simulink model "non_linear_MBS_final"  (R2021b)
settings: Solver=ode15s  StartTime=0  StopTime=30  FixedStep=.00001  RelTol=1e-3
values from neighbouring files (symbols this model uses):
  A_ratio = 1.41    [non_linear_param_MBS_PF_final.m]    (also in non_linear_param_final.m)
  Annulus_area = Bore_area-Rod_area    (= 2356.19449019)    [non_linear_param_MBS_PF_final.m]    (also in non_linear_param_final.m)
  beta_F = 12000e5    (= 1200000000)    [non_linear_param_MBS_PF_final.m]    (also in non_linear_param_final.m)
  Bore_area = pi/4*Bore_diameter^2    (= 3318.30724035)    [non_linear_param_MBS_PF_final.m]    (also in non_linear_param_final.m)
  cyl_c_end = 9e8    (= 900000000)    [non_linear_param_MBS_PF_final.m]    (also in non_linear_param_final.m)
  cyl_c_visc = 1500    [non_linear_param_MBS_PF_final.m]    (also in non_linear_param_final.m)
  cyl_CH_stat = 1/50    (= 0.02)    [non_linear_param_MBS_PF_final.m]    (also in non_linear_param_final.m)
  cyl_F_coul = 75    [non_linear_param_MBS_PF_final.m]    (also in non_linear_param_final.m)
  cyl_FH_stat = 500    [non_linear_param_MBS_PF_final.m]    (also in non_linear_param_final.m)
  cyl_gamma = 250    [non_linear_param_MBS_PF_final.m]    (also in non_linear_param_final.m)
  cyl_k_end = 6e9    (= 6000000000)    [non_linear_param_MBS_PF_final.m]    (also in non_linear_param_final.m)
  cyl_KLi = 0    [non_linear_param_MBS_PF_final.m]    (also in non_linear_param_final.m)
  d_eff_long = sqrt(2*J_sec_long*k_eff_long)    [non_linear_param_MBS_PF_final.m]    (also in non_linear_param_final.m)
  d_eff_short = sqrt(2*J_sec_short*k_eff_short)    [non_linear_param_MBS_PF_final.m]    (also in non_linear_param_final.m)
  DB = 0    [non_linear_param_MBS_PF_final.m]    (also in non_linear_param_final.m = -0.114)
  DR = 1    [non_linear_param_MBS_PF_final.m]    (also in non_linear_param_final.m)
  dr_dcv = 0.7    [non_linear_param_MBS_PF_final.m]    (also in non_linear_param_final.m)
  Eff_Bulk_On = 1    [non_linear_param_MBS_PF_final.m]    (also in non_linear_param_final.m)
  eta_air = 0.7e-2    (= 0.007)    [non_linear_param_MBS_PF_final.m]    (also in non_linear_param_final.m)
  k_cv = 15    [non_linear_param_MBS_PF_final.m]    (also in non_linear_param_final.m)
  k_DCV_PA = 7.85    [non_linear_param_MBS_PF_final.m]    (also in non_linear_param_final.m)
  k_DCV_PB = 7.55    [non_linear_param_MBS_PF_final.m]    (also in non_linear_param_final.m)
  k_DCV_T = 7.85    [non_linear_param_MBS_PF_final.m]    (also in non_linear_param_final.m)
  k_eff_long = E*I/I_sec_long    (= 3050381.94041)    [non_linear_param_MBS_PF_final.m]    (also in non_linear_param_final.m)
  k_eff_short = E*I/I_sec_short    (= 13451432.5427)    [non_linear_param_MBS_PF_final.m]    (also in non_linear_param_final.m)
  k_ocv = 15    [non_linear_param_MBS_PF_final.m]    (also in non_linear_param_final.m)
  K_oil = 1.5e4*0+12000    (= 12000)    [non_linear_param_MBS_PF_final.m]    (also in non_linear_param_final.m)
  k_tanh = 0.005    [non_linear_param_MBS_PF_final.m]    (also in non_linear_param_final.m)
  kappa_air = 1.4    [non_linear_param_MBS_PF_final.m]    (also in non_linear_param_final.m)
  KR = 1    [non_linear_param_MBS_PF_final.m]    (also in non_linear_param_final.m)
  mu_ocv = 10    [non_linear_param_MBS_PF_final.m]    (also in non_linear_param_final.m = 3)
  OL_pa = -0.114    [non_linear_param_MBS_PF_final.m]    (also in non_linear_param_final.m)
  OL_pb = 0.114    [non_linear_param_MBS_PF_final.m]    (also in non_linear_param_final.m)
  p0_A = 62.79    [non_linear_param_MBS_PF_final.m]    (also in non_linear_param_final.m)
  p0_A1 = 1.08    [non_linear_param_MBS_PF_final.m]    (also in non_linear_param_final.m)
  p0_B = 1.213    [non_linear_param_MBS_PF_final.m]    (also in non_linear_param_final.m)
  p_cv_fo = 1    [non_linear_param_MBS_PF_final.m]    (also in non_linear_param_final.m)
  p_cv_set = 2.8    [non_linear_param_MBS_PF_final.m]    (also in non_linear_param_final.m)
  p_ocv_fo = 350    [non_linear_param_MBS_PF_final.m]    (also in non_linear_param_final.m)
  p_ocv_set = 196    [non_linear_param_MBS_PF_final.m]    (also in non_linear_param_final.m = 150)
  p_pc_set = 7    [non_linear_param_MBS_PF_final.m]    (also in non_linear_param_final.m)
  p_R = 1.1    [non_linear_param_MBS_PF_final.m]    (also in non_linear_param_final.m)
  p_S = 180    [non_linear_param_MBS_PF_final.m]    (also in non_linear_param_final.m)
  patm = 1.013e5    (= 101300)    [non_linear_param_MBS_PF_final.m]    (also in non_linear_param_final.m)
  Stroke = 500    [non_linear_param_MBS_PF_final.m]    (also in non_linear_param_final.m)
  t0 = 3    [non_linear_param_MBS_PF_final.m]    (also in non_linear_param_final.m = 1)
  tau_ocv = 0.0889*0+0.001    (= 0.001)    [non_linear_param_MBS_PF_final.m]    (also in non_linear_param_final.m)
  tau_pc = tau_ocv    (= 0.001)    [non_linear_param_MBS_PF_final.m]    (also in non_linear_param_final.m)
  tStepDown = 4.7*0+7.5    (= 7.5)    [non_linear_param_MBS_PF_final.m]    (also in non_linear_param_final.m = 4.6)
  tStepUp = 10.0    (= 10)    [non_linear_param_MBS_PF_final.m]    (also in non_linear_param_final.m = 6.2)
  tWait = 5.0    (= 5)    [non_linear_param_MBS_PF_final.m]    (also in non_linear_param_final.m = 8)
  UL_at = -0.05    [non_linear_param_MBS_PF_final.m]    (also in non_linear_param_final.m)
  UL_bt = 0.05    [non_linear_param_MBS_PF_final.m]    (also in non_linear_param_final.m)
  Up = 1    [non_linear_param_MBS_PF_final.m]    (also in non_linear_param_final.m)
  uS_sp = -0.4    [non_linear_param_MBS_PF_final.m]    (also in non_linear_param_final.m = -0.55)
  V_CH_A = 1.0e-03    (= 0.001)    [non_linear_param_MBS_PF_final.m]    (also in non_linear_param_final.m)
  V_CH_A1 = 0.1e-03    (= 0.0001)    [non_linear_param_MBS_PF_final.m]    (also in non_linear_param_final.m)
  V_CH_B = 1.25e-03    (= 0.00125)    [non_linear_param_MBS_PF_final.m]    (also in non_linear_param_final.m)
  wn_dcv = 30    [non_linear_param_MBS_PF_final.m]    (also in non_linear_param_final.m)
undefined symbols (not defined in the file or in its folder): sigbuilder_block, smiData, uS0, X, Y, Z
libraries: sm_lib x189, nesl_utility x4, eeGeneralControl x2
(part 4 of 6)

== system non_linear_MBS_final/Test/CB_1 ==
  CB_850_1_RIGID : Subsystem
  CB_850_2_RIGID : Subsystem
  CB_plate_1_RIGID : Subsystem
  ReferenceFrame : sm_lib/Frames and Transforms/Reference Frame
  Transform : sm_lib/Frames and Transforms/Rigid Transform  TranslationMethod=Cartesian  TranslationStandardOffset=0  TranslationLengthUnit=mm  TranslationStandardAxis=+Z  TranslationCartesianOffset=smiData.RigidTransform(13).translation  TranslationROffset=0  TranslationROffsetUnits=m  TranslationZOffset=0  TranslationZOffsetUnits=m  TranslationThetaOffset=0  TranslationThetaOffsetUnits=deg  RotationMethod=ArbitraryAxis  RotationAngleUnits=rad  RotationStandardAxis=+Z  RotationAngle=smiData.RigidTransform(13).angle  RotationArbitraryAxis=smiData.RigidTransform(13).axis  FollAlignAxisA=+X  BaseAlignAxisA=+Y  FollAlignAxisB=+Y  BaseAlignAxisB=+Z  RotationSequenceAxes=FollowerAxes  RotationSequence=XYX  RotationSequenceAngles=[0 0 0]  RotationSequenceAnglesUnits=deg  RotationMatrix=[1 0 0; 0 1 0; 0 0 1]
  Transform1 : sm_lib/Frames and Transforms/Rigid Transform  TranslationMethod=Cartesian  TranslationStandardOffset=0  TranslationLengthUnit=mm  TranslationStandardAxis=+Z  TranslationCartesianOffset=smiData.RigidTransform(16).translation  TranslationROffset=0  TranslationROffsetUnits=m  TranslationZOffset=0  TranslationZOffsetUnits=m  TranslationThetaOffset=0  TranslationThetaOffsetUnits=deg  RotationMethod=ArbitraryAxis  RotationAngleUnits=rad  RotationStandardAxis=+Z  RotationAngle=smiData.RigidTransform(16).angle  RotationArbitraryAxis=smiData.RigidTransform(16).axis  FollAlignAxisA=+X  BaseAlignAxisA=+Y  FollAlignAxisB=+Y  BaseAlignAxisB=+Z  RotationSequenceAxes=FollowerAxes  RotationSequence=XYX  RotationSequenceAngles=[0 0 0]  RotationSequenceAnglesUnits=deg  RotationMatrix=[1 0 0; 0 1 0; 0 0 1]
  Transform3 : sm_lib/Frames and Transforms/Rigid Transform  TranslationMethod=Cartesian  TranslationStandardOffset=0  TranslationLengthUnit=mm  TranslationStandardAxis=+Z  TranslationCartesianOffset=smiData.RigidTransform(58).translation  TranslationROffset=0  TranslationROffsetUnits=m  TranslationZOffset=0  TranslationZOffsetUnits=m  TranslationThetaOffset=0  TranslationThetaOffsetUnits=deg  RotationMethod=ArbitraryAxis  RotationAngleUnits=rad  RotationStandardAxis=+Z  RotationAngle=smiData.RigidTransform(58).angle  RotationArbitraryAxis=smiData.RigidTransform(58).axis  FollAlignAxisA=+X  BaseAlignAxisA=+Y  FollAlignAxisB=+Y  BaseAlignAxisB=+Z  RotationSequenceAxes=FollowerAxes  RotationSequence=XYX  RotationSequenceAngles=[0 0 0]  RotationSequenceAnglesUnits=deg  RotationMatrix=[1 0 0; 0 1 0; 0 0 1]
  Transform4 : sm_lib/Frames and Transforms/Rigid Transform  TranslationMethod=Cartesian  TranslationStandardOffset=0  TranslationLengthUnit=mm  TranslationStandardAxis=+Z  TranslationCartesianOffset=smiData.RigidTransform(59).translation  TranslationROffset=0  TranslationROffsetUnits=m  TranslationZOffset=0  TranslationZOffsetUnits=m  TranslationThetaOffset=0  TranslationThetaOffsetUnits=deg  RotationMethod=ArbitraryAxis  RotationAngleUnits=rad  RotationStandardAxis=+Z  RotationAngle=smiData.RigidTransform(59).angle  RotationArbitraryAxis=smiData.RigidTransform(59).axis  FollAlignAxisA=+X  BaseAlignAxisA=+Y  FollAlignAxisB=+Y  BaseAlignAxisB=+Z  RotationSequenceAxes=FollowerAxes  RotationSequence=XYX  RotationSequenceAngles=[0 0 0]  RotationSequenceAnglesUnits=deg  RotationMatrix=[1 0 0; 0 1 0; 0 0 1]
  Transform5 : sm_lib/Frames and Transforms/Rigid Transform  TranslationMethod=Cartesian  TranslationStandardOffset=0  TranslationLengthUnit=mm  TranslationStandardAxis=+Z  TranslationCartesianOffset=smiData.RigidTransform(60).translation  TranslationROffset=0  TranslationROffsetUnits=m  TranslationZOffset=0  TranslationZOffsetUnits=m  TranslationThetaOffset=0  TranslationThetaOffsetUnits=deg  RotationMethod=ArbitraryAxis  RotationAngleUnits=rad  RotationStandardAxis=+Z  RotationAngle=smiData.RigidTransform(60).angle  RotationArbitraryAxis=smiData.RigidTransform(60).axis  FollAlignAxisA=+X  BaseAlignAxisA=+Y  FollAlignAxisB=+Y  BaseAlignAxisB=+Z  RotationSequenceAxes=FollowerAxes  RotationSequence=XYX  RotationSequenceAngles=[0 0 0]  RotationSequenceAnglesUnits=deg  RotationMatrix=[1 0 0; 0 1 0; 0 0 1]
  F1 : PMIOPort  Side=Left
  F : PMIOPort  Port=2  Side=Right
  connections:
  Transform/LConn1 -- ReferenceFrame/RConn1 -- Transform4/LConn1 -- Transform5/LConn1 -- Transform3/LConn1 -- Transform1/LConn1
  Transform/RConn1 -- F/RConn1
  Transform1/RConn1 -- F1/RConn1
  CB_850_1_RIGID/LConn1 -- Transform3/RConn1
  CB_850_2_RIGID/LConn1 -- Transform4/RConn1
  CB_plate_1_RIGID/LConn1 -- Transform5/RConn1

== system non_linear_MBS_final/Test/CB_2 ==
  CB_850_1_RIGID : Subsystem
  CB_850_2_RIGID : Subsystem
  CB_plate_1_RIGID : Subsystem
  ReferenceFrame : sm_lib/Frames and Transforms/Reference Frame
  Rigid Transform4 : sm_lib/Frames and Transforms/Rigid Transform  TranslationMethod=Cartesian  TranslationStandardOffset=0  TranslationLengthUnit=mm  TranslationStandardAxis=+Z  TranslationCartesianOffset=[0 0 -10]  TranslationROffset=0  TranslationROffsetUnits=m  TranslationZOffset=0  TranslationZOffsetUnits=m  TranslationThetaOffset=0  TranslationThetaOffsetUnits=deg  RotationMethod=ArbitraryAxis  RotationAngleUnits=deg  RotationStandardAxis=+Z  RotationAngle=90  RotationArbitraryAxis=[0 0 1]  FollAlignAxisA=+X  BaseAlignAxisA=+Y  FollAlignAxisB=+Y  BaseAlignAxisB=+Z  RotationSequenceAxes=FollowerAxes  RotationSequence=XYX  RotationSequenceAngles=[0 0 0]  RotationSequenceAnglesUnits=deg  RotationMatrix=[1 0 0; 0 1 0; 0 0 1]
  Transform : sm_lib/Frames and Transforms/Rigid Transform  TranslationMethod=Cartesian  TranslationStandardOffset=0  TranslationLengthUnit=mm  TranslationStandardAxis=+Z  TranslationCartesianOffset=smiData.RigidTransform(20).translation  TranslationROffset=0  TranslationROffsetUnits=m  TranslationZOffset=0  TranslationZOffsetUnits=m  TranslationThetaOffset=0  TranslationThetaOffsetUnits=deg  RotationMethod=ArbitraryAxis  RotationAngleUnits=rad  RotationStandardAxis=+Z  RotationAngle=smiData.RigidTransform(20).angle  RotationArbitraryAxis=smiData.RigidTransform(20).axis  FollAlignAxisA=+X  BaseAlignAxisA=+Y  FollAlignAxisB=+Y  BaseAlignAxisB=+Z  RotationSequenceAxes=FollowerAxes  RotationSequence=XYX  RotationSequenceAngles=[0 0 0]  RotationSequenceAnglesUnits=deg  RotationMatrix=[1 0 0; 0 1 0; 0 0 1]
  Transform3 : sm_lib/Frames and Transforms/Rigid Transform  TranslationMethod=Cartesian  TranslationStandardOffset=0  TranslationLengthUnit=mm  TranslationStandardAxis=+Z  TranslationCartesianOffset=smiData.RigidTransform(61).translation  TranslationROffset=0  TranslationROffsetUnits=m  TranslationZOffset=0  TranslationZOffsetUnits=m  TranslationThetaOffset=0  TranslationThetaOffsetUnits=deg  RotationMethod=ArbitraryAxis  RotationAngleUnits=rad  RotationStandardAxis=+Z  RotationAngle=smiData.RigidTransform(61).angle  RotationArbitraryAxis=smiData.RigidTransform(61).axis  FollAlignAxisA=+X  BaseAlignAxisA=+Y  FollAlignAxisB=+Y  BaseAlignAxisB=+Z  RotationSequenceAxes=FollowerAxes  RotationSequence=XYX  RotationSequenceAngles=[0 0 0]  RotationSequenceAnglesUnits=deg  RotationMatrix=[1 0 0; 0 1 0; 0 0 1]
  Transform4 : sm_lib/Frames and Transforms/Rigid Transform  TranslationMethod=Cartesian  TranslationStandardOffset=0  TranslationLengthUnit=mm  TranslationStandardAxis=+Z  TranslationCartesianOffset=smiData.RigidTransform(62).translation  TranslationROffset=0  TranslationROffsetUnits=m  TranslationZOffset=0  TranslationZOffsetUnits=m  TranslationThetaOffset=0  TranslationThetaOffsetUnits=deg  RotationMethod=ArbitraryAxis  RotationAngleUnits=rad  RotationStandardAxis=+Z  RotationAngle=smiData.RigidTransform(62).angle  RotationArbitraryAxis=smiData.RigidTransform(62).axis  FollAlignAxisA=+X  BaseAlignAxisA=+Y  FollAlignAxisB=+Y  BaseAlignAxisB=+Z  RotationSequenceAxes=FollowerAxes  RotationSequence=XYX  RotationSequenceAngles=[0 0 0]  RotationSequenceAnglesUnits=deg  RotationMatrix=[1 0 0; 0 1 0; 0 0 1]
  Transform5 : sm_lib/Frames and Transforms/Rigid Transform  TranslationMethod=Cartesian  TranslationStandardOffset=0  TranslationLengthUnit=mm  TranslationStandardAxis=+Z  TranslationCartesianOffset=smiData.RigidTransform(63).translation  TranslationROffset=0  TranslationROffsetUnits=m  TranslationZOffset=0  TranslationZOffsetUnits=m  TranslationThetaOffset=0  TranslationThetaOffsetUnits=deg  RotationMethod=ArbitraryAxis  RotationAngleUnits=rad  RotationStandardAxis=+Z  RotationAngle=smiData.RigidTransform(63).angle  RotationArbitraryAxis=smiData.RigidTransform(63).axis  FollAlignAxisA=+X  BaseAlignAxisA=+Y  FollAlignAxisB=+Y  BaseAlignAxisB=+Z  RotationSequenceAxes=FollowerAxes  RotationSequence=XYX  RotationSequenceAngles=[0 0 0]  RotationSequenceAnglesUnits=deg  RotationMatrix=[1 0 0; 0 1 0; 0 0 1]
  F1 : PMIOPort  Side=Left
  F : PMIOPort  Port=2  Side=Left
  connections:
  Transform/LConn1 -- Rigid Transform4/LConn1 -- ReferenceFrame/RConn1 -- Transform4/LConn1 -- Transform5/LConn1 -- Transform3/LConn1
  Transform/RConn1 -- F/RConn1
  Rigid Transform4/RConn1 -- F1/RConn1
  CB_850_1_RIGID/LConn1 -- Transform3/RConn1
  CB_plate_1_RIGID/LConn1 -- Transform4/RConn1
  CB_850_2_RIGID/LConn1 -- Transform5/RConn1

== system non_linear_MBS_final/Test/Cylinder Barrel ==
  ReferenceFrame : sm_lib/Frames and Transforms/Reference Frame
  Solid : sm_lib/Body Elements/File Solid  ExtGeomFileName=Cyl_hus_part_Default_sldprt.STEP  UnitType=FromFile  ExtGeomFileUnits=m  StepReaderType=OCC  ExportConvexHullGeometry=off  InertiaType=CalculateFromGeometry  Mass=smiData.Solid(20).mass  MassUnits=kg  CenterOfMass=smiData.Solid(20).CoM  CenterOfMassUnits=mm  MomentsOfInertia=smiData.Solid(20).MoI  MomentsOfInertiaUnits=kg*mm^2  ProductsOfInertia=smiData.Solid(20).PoI  ProductsOfInertiaUnits=kg*mm^2  BasedOnType=Density  Density=8702  DensityUnits=kg/m^3  DoExposeReferenceFrame=on  SerializedFrames=<Frames/>  GraphicType=FromGeometry  MarkerShape=Sphere  MarkerSize=10  GraphicVisPropType=SimpleVisualProperties  GraphicDiffuseColor=[0.0 0.6 1.0]  GraphicSpecularColor=[0.5 0.5 0.5 1.0]  GraphicAmbientColor=[0.15 0.15 0.15 1.0]  GraphicEmissiveColor=[0.0 0.0 0.0 1.0]  GraphicShininess=75  GraphicOpacity=smiData.Solid(20).opacity
  Transform : sm_lib/Frames and Transforms/Rigid Transform  TranslationMethod=Cartesian  TranslationStandardOffset=0  TranslationLengthUnit=mm  TranslationStandardAxis=+Z  TranslationCartesianOffset=smiData.RigidTransform(1).translation  TranslationROffset=0  TranslationROffsetUnits=m  TranslationZOffset=0  TranslationZOffsetUnits=m  TranslationThetaOffset=0  TranslationThetaOffsetUnits=deg  RotationMethod=ArbitraryAxis  RotationAngleUnits=rad  RotationStandardAxis=+Z  RotationAngle=smiData.RigidTransform(1).angle  RotationArbitraryAxis=smiData.RigidTransform(1).axis  FollAlignAxisA=+X  BaseAlignAxisA=+Y  FollAlignAxisB=+Y  BaseAlignAxisB=+Z  RotationSequenceAxes=FollowerAxes  RotationSequence=XYX  RotationSequenceAngles=[0 0 0]  RotationSequenceAnglesUnits=deg  RotationMatrix=[1 0 0; 0 1 0; 0 0 1]
  Transform1 : sm_lib/Frames and Transforms/Rigid Transform  TranslationMethod=Cartesian  TranslationStandardOffset=0  TranslationLengthUnit=mm  TranslationStandardAxis=+Z  TranslationCartesianOffset=smiData.RigidTransform(14).translation  TranslationROffset=0  TranslationROffsetUnits=m  TranslationZOffset=0  TranslationZOffsetUnits=m  TranslationThetaOffset=0  TranslationThetaOffsetUnits=deg  RotationMethod=ArbitraryAxis  RotationAngleUnits=rad  RotationStandardAxis=+Z  RotationAngle=smiData.RigidTransform(14).angle  RotationArbitraryAxis=smiData.RigidTransform(14).axis  FollAlignAxisA=+X  BaseAlignAxisA=+Y  FollAlignAxisB=+Y  BaseAlignAxisB=+Z  RotationSequenceAxes=FollowerAxes  RotationSequence=XYX  RotationSequenceAngles=[0 0 0]  RotationSequenceAnglesUnits=deg  RotationMatrix=[1 0 0; 0 1 0; 0 0 1]
  F1 : PMIOPort  Side=Left
  F : PMIOPort  Port=2  Side=Right
  connections:
  Solid/RConn1 -- ReferenceFrame/RConn1 -- Transform/LConn1 -- Transform1/LConn1
  Transform/RConn1 -- F/RConn1
  Transform1/RConn1 -- F1/RConn1

== system non_linear_MBS_final/Test/Cylinder Rod ==
  ReferenceFrame : sm_lib/Frames and Transforms/Reference Frame
  Solid : sm_lib/Body Elements/File Solid  ExtGeomFileName=Cyl_rod_part_Default_sldprt.STEP  UnitType=FromFile  ExtGeomFileUnits=m  StepReaderType=OCC  ExportConvexHullGeometry=off  InertiaType=CalculateFromGeometry  Mass=5  MassUnits=kg  CenterOfMass=smiData.Solid(1).CoM  CenterOfMassUnits=mm  MomentsOfInertia=smiData.Solid(1).MoI  MomentsOfInertiaUnits=kg*mm^2  ProductsOfInertia=smiData.Solid(1).PoI  ProductsOfInertiaUnits=kg*mm^2  BasedOnType=Mass  Density=1000  DensityUnits=kg/m^3  DoExposeReferenceFrame=on  SerializedFrames=<Frames/>  GraphicType=FromGeometry  MarkerShape=Sphere  MarkerSize=10  GraphicVisPropType=SimpleVisualProperties  GraphicDiffuseColor=smiData.Solid(1).color  GraphicSpecularColor=[0.5 0.5 0.5 1.0]  GraphicAmbientColor=[0.15 0.15 0.15 1.0]  GraphicEmissiveColor=[0.0 0.0 0.0 1.0]  GraphicShininess=75  GraphicOpacity=smiData.Solid(1).opacity
  Transform : sm_lib/Frames and Transforms/Rigid Transform  TranslationMethod=Cartesian  TranslationStandardOffset=0  TranslationLengthUnit=mm  TranslationStandardAxis=+Z  TranslationCartesianOffset=[2.4829027722717001e-12 (-50.259544455820055) 5.8920380567815703e-15]  TranslationROffset=0  TranslationROffsetUnits=m  TranslationZOffset=0  TranslationZOffsetUnits=m  TranslationThetaOffset=0  TranslationThetaOffsetUnits=deg  RotationMethod=ArbitraryAxis  RotationAngleUnits=rad  RotationStandardAxis=+Z  RotationAngle=smiData.RigidTransform(2).angle  RotationArbitraryAxis=smiData.RigidTransform(2).axis  FollAlignAxisA=+X  BaseAlignAxisA=+Y  FollAlignAxisB=+Y  BaseAlignAxisB=+Z  RotationSequenceAxes=FollowerAxes  RotationSequence=XYX  RotationSequenceAngles=[0 0 0]  RotationSequenceAnglesUnits=deg  RotationMatrix=[1 0 0; 0 1 0; 0 0 1]
  Transform1 : sm_lib/Frames and Transforms/Rigid Transform  TranslationMethod=Cartesian  TranslationStandardOffset=0  TranslationLengthUnit=mm  TranslationStandardAxis=+Z  TranslationCartesianOffset=smiData.RigidTransform(19).translation  TranslationROffset=0  TranslationROffsetUnits=m  TranslationZOffset=0  TranslationZOffsetUnits=m  TranslationThetaOffset=0  TranslationThetaOffsetUnits=deg  RotationMethod=ArbitraryAxis  RotationAngleUnits=rad  RotationStandardAxis=+Z  RotationAngle=smiData.RigidTransform(19).angle  RotationArbitraryAxis=smiData.RigidTransform(19).axis  FollAlignAxisA=+X  BaseAlignAxisA=+Y  FollAlignAxisB=+Y  BaseAlignAxisB=+Z  RotationSequenceAxes=FollowerAxes  RotationSequence=XYX  RotationSequenceAngles=[0 0 0]  RotationSequenceAnglesUnits=deg  RotationMatrix=[1 0 0; 0 1 0; 0 0 1]
  F : PMIOPort  Side=Left
  F1 : PMIOPort  Port=2  Side=Right
  connections:
  Solid/RConn1 -- ReferenceFrame/RConn1 -- Transform/LConn1 -- Transform1/LConn1
  Transform1/RConn1 -- F1/RConn1
  Transform/RConn1 -- F/RConn1

== system non_linear_MBS_final/Directional Control Valve/Edge Flows/Flow Characteristics ==
  u_B-T [-] : Inport
  p_B - p_R [bar] : Inport  Port=2
  Abs : Abs
  Constant : Constant  Value=k_DCV_T
  Product6 : Product  Inputs=4  RndMeth=Zero
  Sign : Subsystem
  Sqrt7 : Sqrt  SaturateOnIntegerOverflow=on
  Q_B-T [L/min] : Outport  VectorParamsAs1DForOutWhenUnconnected=off
  wires:
  Sqrt7/1 -> Product6/3  "[bar]^0.5"
  Product6/1 -> Q_B-T [L/min]/1  "[L/min]"
  p_B - p_R [bar]/1 -> Sign/1(In), Abs/1  "[bar]"
  Abs/1 -> Sqrt7/1
  u_B-T [-]/1 -> Product6/2  "[-]"
  Constant/1 -> Product6/1  "[L/min*bar^0.5]"
  Sign/1(Out) -> Product6/4

== system non_linear_MBS_final/Directional Control Valve/Edge Flows/Flow Characteristics1 ==
  u_A-T [-] : Inport
  p_A1 - p_R [bar] : Inport  Port=2
  Abs : Abs
  Constant : Constant  Value=k_DCV_T
  Product6 : Product  Inputs=4  RndMeth=Zero
  Sign : Subsystem
  Sqrt7 : Sqrt  SaturateOnIntegerOverflow=on
  Q_A-T [L/min] : Outport  VectorParamsAs1DForOutWhenUnconnected=off
  wires:
  Sqrt7/1 -> Product6/3  "[bar]^0.5"
  Product6/1 -> Q_A-T [L/min]/1  "[L/min]"
  p_A1 - p_R [bar]/1 -> Sign/1(In), Abs/1  "[bar]"
  Abs/1 -> Sqrt7/1
  u_A-T [-]/1 -> Product6/2  "[-]"
  Sign/1(Out) -> Product6/4
  Constant/1 -> Product6/1  "[L/min*bar^0.5]"

== system non_linear_MBS_final/Directional Control Valve/Edge Flows/Flow Characteristics2 ==
  u_P-A [-] : Inport
  p_C - p_A1 [bar] : Inport  Port=2
  Abs : Abs
  Constant : Constant  Value=k_DCV_PA
  Product6 : Product  Inputs=4  RndMeth=Zero
  Sign : Subsystem
  Sqrt7 : Sqrt  SaturateOnIntegerOverflow=on
  Q_P-A [L/min] : Outport  VectorParamsAs1DForOutWhenUnconnected=off
  wires:
  Sqrt7/1 -> Product6/3  "[bar]^0.5"
  Product6/1 -> Q_P-A [L/min]/1  "[L/min]"
  p_C - p_A1 [bar]/1 -> Sign/1(In), Abs/1  "[bar]"
  Abs/1 -> Sqrt7/1
  u_P-A [-]/1 -> Product6/2  "[-]"
  Sign/1(Out) -> Product6/4
  Constant/1 -> Product6/1  "[L/min*bar^0.5]"

== system non_linear_MBS_final/Directional Control Valve/Edge Flows/Flow Characteristics3 ==
  u_P-B [-] : Inport
  p_C - p_B [bar] : Inport  Port=2
  Abs : Abs
  Constant : Constant  Value=k_DCV_PB
  Product6 : Product  Inputs=4  RndMeth=Zero
  Sign : Subsystem
  Sqrt7 : Sqrt  SaturateOnIntegerOverflow=on
  Q_P-B [L/min] : Outport  VectorParamsAs1DForOutWhenUnconnected=off
  wires:
  Sqrt7/1 -> Product6/3  "[bar]^0.5"
  Product6/1 -> Q_P-B [L/min]/1  "[L/min]"
  p_C - p_B [bar]/1 -> Sign/1(In), Abs/1  "[bar]"
  Abs/1 -> Sqrt7/1
  u_P-B [-]/1 -> Product6/2  "[-]"
  Sign/1(Out) -> Product6/4
  Constant/1 -> Product6/1  "[L/min*bar^0.5]"

== system non_linear_MBS_final/Directional Control Valve/Edge Flows/Q_2 selection ==
  Q_P-A [l/min] : Inport
  Q_P-B [l/min] : Inport  Port=2
  Sum : Sum
  Q_DCV_In [l/min] : Outport  VectorParamsAs1DForOutWhenUnconnected=off
  wires:
  Sum/1 -> Q_DCV_In [l/min]/1
  Q_P-B [l/min]/1 -> Sum/2
  Q_P-A [l/min]/1 -> Sum/1

== system non_linear_MBS_final/Directional Control Valve/Edge Openings/Edge A-T ==
  u_dcv [-] : Inport
  MATLAB Function1 : MATLAB Function  TreatAsAtomicUnit=on
  u_A-T [-] : Outport  VectorParamsAs1DForOutWhenUnconnected=off
  wires:
  MATLAB Function1/1(u_AT) -> u_A-T [-]/1
  u_dcv [-]/1 -> MATLAB Function1/1(u_dcv)

== system non_linear_MBS_final/Directional Control Valve/Edge Openings/Edge B-T ==
  u_dcv [-] : Inport
  MATLAB Function : MATLAB Function  TreatAsAtomicUnit=on
  u_B-T [-] : Outport  VectorParamsAs1DForOutWhenUnconnected=off
  wires:
  u_dcv [-]/1 -> MATLAB Function/1(u_dcv)
  MATLAB Function/1(u_BT) -> u_B-T [-]/1

== system non_linear_MBS_final/Directional Control Valve/Edge Openings/Edge P-A ==
  u_dcv [-] : Inport
  MATLAB Function1 : MATLAB Function  TreatAsAtomicUnit=on
  u_P-A [-] : Outport  VectorParamsAs1DForOutWhenUnconnected=off
  wires:
  u_dcv [-]/1 -> MATLAB Function1/1(u_dcv)
  MATLAB Function1/1(u_PA) -> u_P-A [-]/1

== system non_linear_MBS_final/Directional Control Valve/Edge Openings/Edge P-B ==
  u_dcv [-] : Inport
  MATLAB Function1 : MATLAB Function  TreatAsAtomicUnit=on
  u_P-B [-] : Outport  VectorParamsAs1DForOutWhenUnconnected=off
  wires:
  u_dcv [-]/1 -> MATLAB Function1/1(u_dcv)
  MATLAB Function1/1(u_PB) -> u_P-B [-]/1

== system non_linear_MBS_final/Over-Centre Valve/Capasitance/Bulk Modulus ==
  Bulk/Volume : Constant  Value=K_oil
  Eff_Bulk_On : Constant  Value=Eff_Bulk_On
  Effective Bulk Modulus : Subsystem
  Switch : Switch  Criteria=u2 > Threshold
  K_oil : Outport  VectorParamsAs1DForOutWhenUnconnected=off
  wires:
  Bulk/Volume/1 -> Switch/3  "[bar]"
  Switch/1 -> K_oil/1
  Eff_Bulk_On/1 -> Switch/2
  Effective Bulk Modulus/1(K_oil_eff) -> Switch/1  "[bar]"

== system non_linear_MBS_final/Over-Centre Valve/Check Valve/Sign ==
  In : Inport
  Gain2 : Gain  Gain=1/k_tanh
  Trigonometric Function : Trigonometry  Operator=tanh
  Out : Outport  VectorParamsAs1DForOutWhenUnconnected=off
  wires:
  In/1 -> Gain2/1
  Gain2/1 -> Trigonometric Function/1
  Trigonometric Function/1 -> Out/1  "[-]"

== system non_linear_MBS_final/Over-Centre Valve/Over-Centre Valve/Sign ==
  In : Inport
  Gain2 : Gain  Gain=1/k_tanh
  Trigonometric Function : Trigonometry  Operator=tanh
  Out : Outport  VectorParamsAs1DForOutWhenUnconnected=off
  wires:
  In/1 -> Gain2/1
  Gain2/1 -> Trigonometric Function/1
  Trigonometric Function/1 -> Out/1  "[-]"

== system non_linear_MBS_final/Subsystem/CH Bore Side/Bulk Modulus ==
  Bulk/Volume : Constant  Value=K_oil
  Eff_Bulk_On : Constant  Value=Eff_Bulk_On
  Effective Bulk Modulus : Subsystem
  Switch : Switch  Criteria=u2 > Threshold
  K_oil : Outport  VectorParamsAs1DForOutWhenUnconnected=off
  wires:
  Bulk/Volume/1 -> Switch/3  "[bar]"
  Switch/1 -> K_oil/1
  Eff_Bulk_On/1 -> Switch/2
  Effective Bulk Modulus/1(K_oil_eff) -> Switch/1  "[bar]"

== system non_linear_MBS_final/Subsystem/CH Rod Side/Bulk Modulus ==
  Bulk/Volume : Constant  Value=K_oil
  Eff_Bulk_On : Constant  Value=Eff_Bulk_On
  Effective Bulk Modulus : Subsystem
  Switch : Switch  Criteria=u2 > Threshold
  K_oil : Outport  VectorParamsAs1DForOutWhenUnconnected=off
  wires:
  Bulk/Volume/1 -> Switch/3  "[bar]"
  Switch/1 -> K_oil/1
  Eff_Bulk_On/1 -> Switch/2
  Effective Bulk Modulus/1(K_oil_eff) -> Switch/1  "[bar]"

== system non_linear_MBS_final/Subsystem/Cylinder forces/Lower Stroke Limit ==
  x_cyl [m] : Inport
  v_cyl [m] : Inport  Port=2
  Abs : Abs
  Add1 : Sum
  Constant : Constant  Value=0
  Constant1 : Constant  Value=0
  End stroke spring coeff : Constant  Value=cyl_k_end
  End stroke viscous coeff : Constant  Value=cyl_c_end
  Gain : Gain  Gain=-1
  Saturation : Saturate  UpperLimit=0  LowerLimit=-inf
  Switch : Switch  Criteria=u2 > Threshold
  Switch1 : Switch  Criteria=u2 > Threshold
  mult : Product  Inputs=**
  mult1 : Product  Inputs=**
  F_cyl_contact [N] : Outport  VectorParamsAs1DForOutWhenUnconnected=off
  wires:
  Add1/1 -> F_cyl_contact [N]/1
  mult1/1 -> Saturation/1
  mult/1 -> Add1/1
  Switch1/1 -> Gain/1
  Abs/1 -> mult/2
  Switch/1 -> mult/1
  x_cyl [m]/1 -> Switch1/2, Abs/1, Switch/2
  Constant/1 -> Switch/1
  End stroke spring coeff/1 -> Switch/3
  End stroke viscous coeff/1 -> Switch1/3
  Constant1/1 -> Switch1/1
  Gain/1 -> mult1/1
  v_cyl [m]/1 -> mult1/2
  Saturation/1 -> Add1/2

== system non_linear_MBS_final/Subsystem/Cylinder forces/Stribeck Friction1 ==
  v_cyl [m/s] : Inport
  Mux : Mux  Inputs=6
  Stribeck Curve : Fcn  Expr=u(1)*u(2)+tanh(u(1)*u(3))*(u(4)+u(5)*exp(-(u(1)*tanh(u(1)*u(3)))/u(6)))
  "coefficient of viscous friction " : Constant  Value=cyl_c_visc
  coulomb friction : Constant  Value=cyl_F_coul
  friction force approximation constant : Constant  Value=cyl_gamma
  friction time constant : Constant  Value=cyl_CH_stat
  static friction : Constant  Value=cyl_FH_stat
  F [N] : Outport  VectorParamsAs1DForOutWhenUnconnected=off
  wires:
  v_cyl [m/s]/1 -> Mux/1
  Stribeck Curve/1 -> F [N]/1
  friction time constant/1 -> Mux/6
  static friction/1 -> Mux/5
  coulomb friction/1 -> Mux/4
  friction force approximation constant/1 -> Mux/3
  "coefficient of viscous friction "/1 -> Mux/2
  Mux/1 -> Stribeck Curve/1

== system non_linear_MBS_final/Subsystem/Cylinder forces/Upper Stroke Limit ==
  x_cyl [m] : Inport
  v_cyl [m/s] : Inport  Port=2
  Add : Sum  Inputs=|+-
  Add1 : Sum
  Constant : Constant  Value=0
  Constant1 : Constant  Value=0
  End stroke spring coeff : Constant  Value=cyl_k_end
  End stroke viscous coeff : Constant  Value=cyl_c_end
  Gain : Gain  Gain=1/1000
  Saturation : Saturate  UpperLimit=inf  LowerLimit=0
  Stroke Length : Constant  Value=Stroke
  Switch : Switch  Criteria=u2 > Threshold  Threshold=Stroke/1000
  Switch1 : Switch  Criteria=u2 > Threshold  Threshold=Stroke/1000
  mult : Product  Inputs=**
  mult1 : Product  Inputs=**
  F_cyl_contact [N] : Outport  VectorParamsAs1DForOutWhenUnconnected=off
  wires:
  x_cyl [m]/1 -> Add/1, Switch1/2, Switch/2
  End stroke spring coeff/1 -> Switch/1
  Constant/1 -> Switch/3
  Stroke Length/1 -> Gain/1
  Switch/1 -> mult/1
  Add/1 -> mult/2
  End stroke viscous coeff/1 -> Switch1/1
  Constant1/1 -> Switch1/3
  Switch1/1 -> mult1/1
  mult/1 -> Add1/1
  mult1/1 -> Saturation/1
  Add1/1 -> F_cyl_contact [N]/1
  v_cyl [m/s]/1 -> mult1/2
  Saturation/1 -> Add1/2
  Gain/1 -> Add/2  "[m]"

== system non_linear_MBS_final/Test/Base/Base_bearing_mount_1_RIGID ==
  Base_bearing_mount_sideways_1_RIGID : Subsystem
  Base_bearing_mount_support_1_RIGID : Subsystem
  Base_bearing_mount_support_2_RIGID : Subsystem
  ReferenceFrame : sm_lib/Frames and Transforms/Reference Frame
  Transform : sm_lib/Frames and Transforms/Rigid Transform  TranslationMethod=Cartesian  TranslationStandardOffset=0  TranslationLengthUnit=mm  TranslationStandardAxis=+Z  TranslationCartesianOffset=smiData.RigidTransform(29).translation  TranslationROffset=0  TranslationROffsetUnits=m  TranslationZOffset=0  TranslationZOffsetUnits=m  TranslationThetaOffset=0  TranslationThetaOffsetUnits=deg  RotationMethod=ArbitraryAxis  RotationAngleUnits=rad  RotationStandardAxis=+Z  RotationAngle=smiData.RigidTransform(29).angle  RotationArbitraryAxis=smiData.RigidTransform(29).axis  FollAlignAxisA=+X  BaseAlignAxisA=+Y  FollAlignAxisB=+Y  BaseAlignAxisB=+Z  RotationSequenceAxes=FollowerAxes  RotationSequence=XYX  RotationSequenceAngles=[0 0 0]  RotationSequenceAnglesUnits=deg  RotationMatrix=[1 0 0; 0 1 0; 0 0 1]
  Transform1 : sm_lib/Frames and Transforms/Rigid Transform  TranslationMethod=Cartesian  TranslationStandardOffset=0  TranslationLengthUnit=mm  TranslationStandardAxis=+Z  TranslationCartesianOffset=smiData.RigidTransform(30).translation  TranslationROffset=0  TranslationROffsetUnits=m  TranslationZOffset=0  TranslationZOffsetUnits=m  TranslationThetaOffset=0  TranslationThetaOffsetUnits=deg  RotationMethod=ArbitraryAxis  RotationAngleUnits=rad  RotationStandardAxis=+Z  RotationAngle=smiData.RigidTransform(30).angle  RotationArbitraryAxis=smiData.RigidTransform(30).axis  FollAlignAxisA=+X  BaseAlignAxisA=+Y  FollAlignAxisB=+Y  BaseAlignAxisB=+Z  RotationSequenceAxes=FollowerAxes  RotationSequence=XYX  RotationSequenceAngles=[0 0 0]  RotationSequenceAnglesUnits=deg  RotationMatrix=[1 0 0; 0 1 0; 0 0 1]
  Transform2 : sm_lib/Frames and Transforms/Rigid Transform  TranslationMethod=Cartesian  TranslationStandardOffset=0  TranslationLengthUnit=mm  TranslationStandardAxis=+Z  TranslationCartesianOffset=smiData.RigidTransform(31).translation  TranslationROffset=0  TranslationROffsetUnits=m  TranslationZOffset=0  TranslationZOffsetUnits=m  TranslationThetaOffset=0  TranslationThetaOffsetUnits=deg  RotationMethod=ArbitraryAxis  RotationAngleUnits=rad  RotationStandardAxis=+Z  RotationAngle=smiData.RigidTransform(31).angle  RotationArbitraryAxis=smiData.RigidTransform(31).axis  FollAlignAxisA=+X  BaseAlignAxisA=+Y  FollAlignAxisB=+Y  BaseAlignAxisB=+Z  RotationSequenceAxes=FollowerAxes  RotationSequence=XYX  RotationSequenceAngles=[0 0 0]  RotationSequenceAnglesUnits=deg  RotationMatrix=[1 0 0; 0 1 0; 0 0 1]
  Transform3 : sm_lib/Frames and Transforms/Rigid Transform  TranslationMethod=Cartesian  TranslationStandardOffset=0  TranslationLengthUnit=mm  TranslationStandardAxis=+Z  TranslationCartesianOffset=smiData.RigidTransform(32).translation  TranslationROffset=0  TranslationROffsetUnits=m  TranslationZOffset=0  TranslationZOffsetUnits=m  TranslationThetaOffset=0  TranslationThetaOffsetUnits=deg  RotationMethod=ArbitraryAxis  RotationAngleUnits=rad  RotationStandardAxis=+Z  RotationAngle=smiData.RigidTransform(32).angle  RotationArbitraryAxis=smiData.RigidTransform(32).axis  FollAlignAxisA=+X  BaseAlignAxisA=+Y  FollAlignAxisB=+Y  BaseAlignAxisB=+Z  RotationSequenceAxes=FollowerAxes  RotationSequence=XYX  RotationSequenceAngles=[0 0 0]  RotationSequenceAnglesUnits=deg  RotationMatrix=[1 0 0; 0 1 0; 0 0 1]
  F : PMIOPort  Side=Right
  connections:
  Base_bearing_mount_sideways_1_RIGID/LConn1 -- Transform/RConn1
  Base_bearing_mount_support_2_RIGID/LConn1 -- Transform1/RConn1
  Base_bearing_mount_support_1_RIGID/LConn1 -- Transform2/RConn1
  Transform/LConn1 -- ReferenceFrame/RConn1 -- Transform1/LConn1 -- Transform2/LConn1 -- Transform3/RConn1
  Transform3/LConn1 -- F/RConn1

== system non_linear_MBS_final/Test/Base/Base_bottomplate_1_RIGID ==
  ReferenceFrame : sm_lib/Frames and Transforms/Reference Frame
  Solid : sm_lib/Body Elements/File Solid  ExtGeomFileName=Base_bottomplate_Default_sldprt.STEP  UnitType=FromFile  ExtGeomFileUnits=m  StepReaderType=HEX  ExportConvexHullGeometry=off  InertiaType=Custom  Mass=smiData.Solid(13).mass  MassUnits=kg  CenterOfMass=smiData.Solid(13).CoM  CenterOfMassUnits=mm  MomentsOfInertia=smiData.Solid(13).MoI  MomentsOfInertiaUnits=kg*mm^2  ProductsOfInertia=smiData.Solid(13).PoI  ProductsOfInertiaUnits=kg*mm^2  BasedOnType=Density  Density=1000  DensityUnits=kg/m^3  DoExposeReferenceFrame=on  SerializedFrames=<Frames/>  GraphicType=FromGeometry  MarkerShape=Sphere  MarkerSize=10  GraphicVisPropType=SimpleVisualProperties  GraphicDiffuseColor=smiData.Solid(13).color  GraphicSpecularColor=[0.5 0.5 0.5 1.0]  GraphicAmbientColor=[0.15 0.15 0.15 1.0]  GraphicEmissiveColor=[0.0 0.0 0.0 1.0]  GraphicShininess=75  GraphicOpacity=smiData.Solid(13).opacity
  F : PMIOPort  Side=Left
  connections:
  Solid/RConn1 -- ReferenceFrame/RConn1 -- F/RConn1

== system non_linear_MBS_final/Test/Base/Base_profil_base_along_arm_1_RIGID ==
  ReferenceFrame : sm_lib/Frames and Transforms/Reference Frame
  Solid : sm_lib/Body Elements/File Solid  ExtGeomFileName=Base_profil_base_along_arm_Default_sldprt.STEP  UnitType=FromFile  ExtGeomFileUnits=m  StepReaderType=HEX  ExportConvexHullGeometry=off  InertiaType=Custom  Mass=smiData.Solid(4).mass  MassUnits=kg  CenterOfMass=smiData.Solid(4).CoM  CenterOfMassUnits=mm  MomentsOfInertia=smiData.Solid(4).MoI  MomentsOfInertiaUnits=kg*mm^2  ProductsOfInertia=smiData.Solid(4).PoI  ProductsOfInertiaUnits=kg*mm^2  BasedOnType=Density  Density=1000  DensityUnits=kg/m^3  DoExposeReferenceFrame=on  SerializedFrames=<Frames/>  GraphicType=FromGeometry  MarkerShape=Sphere  MarkerSize=10  GraphicVisPropType=SimpleVisualProperties  GraphicDiffuseColor=smiData.Solid(4).color  GraphicSpecularColor=[0.5 0.5 0.5 1.0]  GraphicAmbientColor=[0.15 0.15 0.15 1.0]  GraphicEmissiveColor=[0.0 0.0 0.0 1.0]  GraphicShininess=75  GraphicOpacity=smiData.Solid(4).opacity
  F : PMIOPort  Side=Left
  connections:
  Solid/RConn1 -- ReferenceFrame/RConn1 -- F/RConn1

== system non_linear_MBS_final/Test/Base/Base_profil_base_along_arm_2_RIGID ==
  ReferenceFrame : sm_lib/Frames and Transforms/Reference Frame
  Solid : sm_lib/Body Elements/File Solid  ExtGeomFileName=Base_profil_base_along_arm_Default_sldprt.STEP  UnitType=FromFile  ExtGeomFileUnits=m  StepReaderType=HEX  ExportConvexHullGeometry=off  InertiaType=Custom  Mass=smiData.Solid(4).mass  MassUnits=kg  CenterOfMass=smiData.Solid(4).CoM  CenterOfMassUnits=mm  MomentsOfInertia=smiData.Solid(4).MoI  MomentsOfInertiaUnits=kg*mm^2  ProductsOfInertia=smiData.Solid(4).PoI  ProductsOfInertiaUnits=kg*mm^2  BasedOnType=Density  Density=1000  DensityUnits=kg/m^3  DoExposeReferenceFrame=on  SerializedFrames=<Frames/>  GraphicType=FromGeometry  MarkerShape=Sphere  MarkerSize=10  GraphicVisPropType=SimpleVisualProperties  GraphicDiffuseColor=smiData.Solid(4).color  GraphicSpecularColor=[0.5 0.5 0.5 1.0]  GraphicAmbientColor=[0.15 0.15 0.15 1.0]  GraphicEmissiveColor=[0.0 0.0 0.0 1.0]  GraphicShininess=75  GraphicOpacity=smiData.Solid(4).opacity
  F : PMIOPort  Side=Left
  connections:
  Solid/RConn1 -- ReferenceFrame/RConn1 -- F/RConn1

== system non_linear_MBS_final/Test/Base/Base_profil_base_sideways_1_RIGID ==
  ReferenceFrame : sm_lib/Frames and Transforms/Reference Frame
  Solid : sm_lib/Body Elements/File Solid  ExtGeomFileName=Base_profil_base_sideways_Default_sldprt.STEP  UnitType=FromFile  ExtGeomFileUnits=m  StepReaderType=HEX  ExportConvexHullGeometry=off  InertiaType=Custom  Mass=smiData.Solid(9).mass  MassUnits=kg  CenterOfMass=smiData.Solid(9).CoM  CenterOfMassUnits=mm  MomentsOfInertia=smiData.Solid(9).MoI  MomentsOfInertiaUnits=kg*mm^2  ProductsOfInertia=smiData.Solid(9).PoI  ProductsOfInertiaUnits=kg*mm^2  BasedOnType=Density  Density=1000  DensityUnits=kg/m^3  DoExposeReferenceFrame=on  SerializedFrames=<Frames/>  GraphicType=FromGeometry  MarkerShape=Sphere  MarkerSize=10  GraphicVisPropType=SimpleVisualProperties  GraphicDiffuseColor=smiData.Solid(9).color  GraphicSpecularColor=[0.5 0.5 0.5 1.0]  GraphicAmbientColor=[0.15 0.15 0.15 1.0]  GraphicEmissiveColor=[0.0 0.0 0.0 1.0]  GraphicShininess=75  GraphicOpacity=smiData.Solid(9).opacity
  F : PMIOPort  Side=Left
  connections:
  Solid/RConn1 -- ReferenceFrame/RConn1 -- F/RConn1

== system non_linear_MBS_final/Test/Base/Base_profil_base_sideways_2_RIGID ==
  ReferenceFrame : sm_lib/Frames and Transforms/Reference Frame
  Solid : sm_lib/Body Elements/File Solid  ExtGeomFileName=Base_profil_base_sideways_Default_sldprt.STEP  UnitType=FromFile  ExtGeomFileUnits=m  StepReaderType=HEX  ExportConvexHullGeometry=off  InertiaType=Custom  Mass=smiData.Solid(9).mass  MassUnits=kg  CenterOfMass=smiData.Solid(9).CoM  CenterOfMassUnits=mm  MomentsOfInertia=smiData.Solid(9).MoI  MomentsOfInertiaUnits=kg*mm^2  ProductsOfInertia=smiData.Solid(9).PoI  ProductsOfInertiaUnits=kg*mm^2  BasedOnType=Density  Density=1000  DensityUnits=kg/m^3  DoExposeReferenceFrame=on  SerializedFrames=<Frames/>  GraphicType=FromGeometry  MarkerShape=Sphere  MarkerSize=10  GraphicVisPropType=SimpleVisualProperties  GraphicDiffuseColor=smiData.Solid(9).color  GraphicSpecularColor=[0.5 0.5 0.5 1.0]  GraphicAmbientColor=[0.15 0.15 0.15 1.0]  GraphicEmissiveColor=[0.0 0.0 0.0 1.0]  GraphicShininess=75  GraphicOpacity=smiData.Solid(9).opacity
  F : PMIOPort  Side=Left
  connections:
  Solid/RConn1 -- ReferenceFrame/RConn1 -- F/RConn1

== system non_linear_MBS_final/Test/Base/Base_profil_base_support_1_RIGID ==
  ReferenceFrame : sm_lib/Frames and Transforms/Reference Frame
  Solid : sm_lib/Body Elements/File Solid  ExtGeomFileName=Base_profil_base_support_Default_sldprt.STEP  UnitType=FromFile  ExtGeomFileUnits=m  StepReaderType=HEX  ExportConvexHullGeometry=off  InertiaType=Custom  Mass=smiData.Solid(3).mass  MassUnits=kg  CenterOfMass=smiData.Solid(3).CoM  CenterOfMassUnits=mm  MomentsOfInertia=smiData.Solid(3).MoI  MomentsOfInertiaUnits=kg*mm^2  ProductsOfInertia=smiData.Solid(3).PoI  ProductsOfInertiaUnits=kg*mm^2  BasedOnType=Density  Density=1000  DensityUnits=kg/m^3  DoExposeReferenceFrame=on  SerializedFrames=<Frames/>  GraphicType=FromGeometry  MarkerShape=Sphere  MarkerSize=10  GraphicVisPropType=SimpleVisualProperties  GraphicDiffuseColor=smiData.Solid(3).color  GraphicSpecularColor=[0.5 0.5 0.5 1.0]  GraphicAmbientColor=[0.15 0.15 0.15 1.0]  GraphicEmissiveColor=[0.0 0.0 0.0 1.0]  GraphicShininess=75  GraphicOpacity=smiData.Solid(3).opacity
  F : PMIOPort  Side=Left
  connections:
  Solid/RConn1 -- ReferenceFrame/RConn1 -- F/RConn1

== system non_linear_MBS_final/Test/Base/Base_profil_base_support_2_1_RIGID ==
  ReferenceFrame : sm_lib/Frames and Transforms/Reference Frame
  Solid : sm_lib/Body Elements/File Solid  ExtGeomFileName=Base_profil_base_support_2_Default_sldprt.STEP  UnitType=FromFile  ExtGeomFileUnits=m  StepReaderType=HEX  ExportConvexHullGeometry=off  InertiaType=Custom  Mass=smiData.Solid(5).mass  MassUnits=kg  CenterOfMass=smiData.Solid(5).CoM  CenterOfMassUnits=mm  MomentsOfInertia=smiData.Solid(5).MoI  MomentsOfInertiaUnits=kg*mm^2  ProductsOfInertia=smiData.Solid(5).PoI  ProductsOfInertiaUnits=kg*mm^2  BasedOnType=Density  Density=1000  DensityUnits=kg/m^3  DoExposeReferenceFrame=on  SerializedFrames=<Frames/>  GraphicType=FromGeometry  MarkerShape=Sphere  MarkerSize=10  GraphicVisPropType=SimpleVisualProperties  GraphicDiffuseColor=smiData.Solid(5).color  GraphicSpecularColor=[0.5 0.5 0.5 1.0]  GraphicAmbientColor=[0.15 0.15 0.15 1.0]  GraphicEmissiveColor=[0.0 0.0 0.0 1.0]  GraphicShininess=75  GraphicOpacity=smiData.Solid(5).opacity
  F : PMIOPort  Side=Left
  connections:
  Solid/RConn1 -- ReferenceFrame/RConn1 -- F/RConn1

== system non_linear_MBS_final/Test/Base/Base_profil_cyl_mount1_1_RIGID ==
  ReferenceFrame : sm_lib/Frames and Transforms/Reference Frame
  Solid : sm_lib/Body Elements/File Solid  ExtGeomFileName=Base_profil_cyl_mount1_Default_sldprt.STEP  UnitType=FromFile  ExtGeomFileUnits=m  StepReaderType=HEX  ExportConvexHullGeometry=off  InertiaType=Custom  Mass=smiData.Solid(11).mass  MassUnits=kg  CenterOfMass=smiData.Solid(11).CoM  CenterOfMassUnits=mm  MomentsOfInertia=smiData.Solid(11).MoI  MomentsOfInertiaUnits=kg*mm^2  ProductsOfInertia=smiData.Solid(11).PoI  ProductsOfInertiaUnits=kg*mm^2  BasedOnType=Density  Density=1000  DensityUnits=kg/m^3  DoExposeReferenceFrame=on  SerializedFrames=<Frames/>  GraphicType=FromGeometry  MarkerShape=Sphere  MarkerSize=10  GraphicVisPropType=SimpleVisualProperties  GraphicDiffuseColor=smiData.Solid(11).color  GraphicSpecularColor=[0.5 0.5 0.5 1.0]  GraphicAmbientColor=[0.15 0.15 0.15 1.0]  GraphicEmissiveColor=[0.0 0.0 0.0 1.0]  GraphicShininess=75  GraphicOpacity=smiData.Solid(11).opacity
  F : PMIOPort  Side=Left
  connections:
  Solid/RConn1 -- ReferenceFrame/RConn1 -- F/RConn1

== system non_linear_MBS_final/Test/Base/Base_profil_cyl_mount_support_sideways_1_RIGID ==
  ReferenceFrame : sm_lib/Frames and Transforms/Reference Frame
  Solid : sm_lib/Body Elements/File Solid  ExtGeomFileName=Base_profil_cyl_mount_support_sideways_Default_sldprt.STEP  UnitType=FromFile  ExtGeomFileUnits=m  StepReaderType=HEX  ExportConvexHullGeometry=off  InertiaType=Custom  Mass=smiData.Solid(2).mass  MassUnits=kg  CenterOfMass=smiData.Solid(2).CoM  CenterOfMassUnits=mm  MomentsOfInertia=smiData.Solid(2).MoI  MomentsOfInertiaUnits=kg*mm^2  ProductsOfInertia=smiData.Solid(2).PoI  ProductsOfInertiaUnits=kg*mm^2  BasedOnType=Density  Density=1000  DensityUnits=kg/m^3  DoExposeReferenceFrame=on  SerializedFrames=<Frames/>  GraphicType=FromGeometry  MarkerShape=Sphere  MarkerSize=10  GraphicVisPropType=SimpleVisualProperties  GraphicDiffuseColor=smiData.Solid(2).color  GraphicSpecularColor=[0.5 0.5 0.5 1.0]  GraphicAmbientColor=[0.15 0.15 0.15 1.0]  GraphicEmissiveColor=[0.0 0.0 0.0 1.0]  GraphicShininess=75  GraphicOpacity=smiData.Solid(2).opacity
  F : PMIOPort  Side=Left
  connections:
  Solid/RConn1 -- ReferenceFrame/RConn1 -- F/RConn1

== system non_linear_MBS_final/Test/Base/Base_profil_incline_1_RIGID ==
  ReferenceFrame : sm_lib/Frames and Transforms/Reference Frame
  Solid : sm_lib/Body Elements/File Solid  ExtGeomFileName=Base_profil_incline_Default_sldprt.STEP  UnitType=FromFile  ExtGeomFileUnits=m  StepReaderType=HEX  ExportConvexHullGeometry=off  InertiaType=Custom  Mass=smiData.Solid(10).mass  MassUnits=kg  CenterOfMass=smiData.Solid(10).CoM  CenterOfMassUnits=mm  MomentsOfInertia=smiData.Solid(10).MoI  MomentsOfInertiaUnits=kg*mm^2  ProductsOfInertia=smiData.Solid(10).PoI  ProductsOfInertiaUnits=kg*mm^2  BasedOnType=Density  Density=1000  DensityUnits=kg/m^3  DoExposeReferenceFrame=on  SerializedFrames=<Frames/>  GraphicType=FromGeometry  MarkerShape=Sphere  MarkerSize=10  GraphicVisPropType=SimpleVisualProperties  GraphicDiffuseColor=smiData.Solid(10).color  GraphicSpecularColor=[0.5 0.5 0.5 1.0]  GraphicAmbientColor=[0.15 0.15 0.15 1.0]  GraphicEmissiveColor=[0.0 0.0 0.0 1.0]  GraphicShininess=75  GraphicOpacity=smiData.Solid(10).opacity
  F : PMIOPort  Side=Left
  connections:
  Solid/RConn1 -- ReferenceFrame/RConn1 -- F/RConn1

== system non_linear_MBS_final/Test/Base/Base_profil_incline_2_RIGID ==
  ReferenceFrame : sm_lib/Frames and Transforms/Reference Frame
  Solid : sm_lib/Body Elements/File Solid  ExtGeomFileName=Base_profil_incline_Default_sldprt.STEP  UnitType=FromFile  ExtGeomFileUnits=m  StepReaderType=HEX  ExportConvexHullGeometry=off  InertiaType=Custom  Mass=smiData.Solid(10).mass  MassUnits=kg  CenterOfMass=smiData.Solid(10).CoM  CenterOfMassUnits=mm  MomentsOfInertia=smiData.Solid(10).MoI  MomentsOfInertiaUnits=kg*mm^2  ProductsOfInertia=smiData.Solid(10).PoI  ProductsOfInertiaUnits=kg*mm^2  BasedOnType=Density  Density=1000  DensityUnits=kg/m^3  DoExposeReferenceFrame=on  SerializedFrames=<Frames/>  GraphicType=FromGeometry  MarkerShape=Sphere  MarkerSize=10  GraphicVisPropType=SimpleVisualProperties  GraphicDiffuseColor=smiData.Solid(10).color  GraphicSpecularColor=[0.5 0.5 0.5 1.0]  GraphicAmbientColor=[0.15 0.15 0.15 1.0]  GraphicEmissiveColor=[0.0 0.0 0.0 1.0]  GraphicShininess=75  GraphicOpacity=smiData.Solid(10).opacity
  F : PMIOPort  Side=Left
  connections:
  Solid/RConn1 -- ReferenceFrame/RConn1 -- F/RConn1

== system non_linear_MBS_final/Test/Base/Base_profil_tower_1_RIGID ==
  ReferenceFrame : sm_lib/Frames and Transforms/Reference Frame
  Solid : sm_lib/Body Elements/File Solid  ExtGeomFileName=Base_profil_tower_Default_sldprt.STEP  UnitType=FromFile  ExtGeomFileUnits=m  StepReaderType=HEX  ExportConvexHullGeometry=off  InertiaType=Custom  Mass=smiData.Solid(8).mass  MassUnits=kg  CenterOfMass=smiData.Solid(8).CoM  CenterOfMassUnits=mm  MomentsOfInertia=smiData.Solid(8).MoI  MomentsOfInertiaUnits=kg*mm^2  ProductsOfInertia=smiData.Solid(8).PoI  ProductsOfInertiaUnits=kg*mm^2  BasedOnType=Density  Density=1000  DensityUnits=kg/m^3  DoExposeReferenceFrame=on  SerializedFrames=<Frames/>  GraphicType=FromGeometry  MarkerShape=Sphere  MarkerSize=10  GraphicVisPropType=SimpleVisualProperties  GraphicDiffuseColor=smiData.Solid(8).color  GraphicSpecularColor=[0.5 0.5 0.5 1.0]  GraphicAmbientColor=[0.15 0.15 0.15 1.0]  GraphicEmissiveColor=[0.0 0.0 0.0 1.0]  GraphicShininess=75  GraphicOpacity=smiData.Solid(8).opacity
  F : PMIOPort  Side=Left
  connections:
  Solid/RConn1 -- ReferenceFrame/RConn1 -- F/RConn1

== system non_linear_MBS_final/Test/Base/Base_profil_tower_2_RIGID ==
  ReferenceFrame : sm_lib/Frames and Transforms/Reference Frame
  Solid : sm_lib/Body Elements/File Solid  ExtGeomFileName=Base_profil_tower_Default_sldprt.STEP  UnitType=FromFile  ExtGeomFileUnits=m  StepReaderType=HEX  ExportConvexHullGeometry=off  InertiaType=Custom  Mass=smiData.Solid(8).mass  MassUnits=kg  CenterOfMass=smiData.Solid(8).CoM  CenterOfMassUnits=mm  MomentsOfInertia=smiData.Solid(8).MoI  MomentsOfInertiaUnits=kg*mm^2  ProductsOfInertia=smiData.Solid(8).PoI  ProductsOfInertiaUnits=kg*mm^2  BasedOnType=Density  Density=1000  DensityUnits=kg/m^3  DoExposeReferenceFrame=on  SerializedFrames=<Frames/>  GraphicType=FromGeometry  MarkerShape=Sphere  MarkerSize=10  GraphicVisPropType=SimpleVisualProperties  GraphicDiffuseColor=smiData.Solid(8).color  GraphicSpecularColor=[0.5 0.5 0.5 1.0]  GraphicAmbientColor=[0.15 0.15 0.15 1.0]  GraphicEmissiveColor=[0.0 0.0 0.0 1.0]  GraphicShininess=75  GraphicOpacity=smiData.Solid(8).opacity
  F : PMIOPort  Side=Left
  connections:
  Solid/RConn1 -- ReferenceFrame/RConn1 -- F/RConn1
